simulink library "inp"  (Simulink 8.2)
settings: Solver=ode45  SolverMode=SingleTasking  StartTime=0.0  StopTime=10.0  RelTol=1e-3
undefined symbols (not defined in the file or in its folder): C_TICK_TIME_S, ENUM_FAULTSTATE_FAIL, ENUM_FAULTSTATE_INDETERMINATE, ENUM_FAULTSTATE_PASS, ENUM_KEYSWITCH_ON, FALSE, KINP_ABS1CANCommuOk_flg_ovrdflg, KINP_ABS1CANCommuOk_flg_ovrdval, KINP_ABS1CANPeriodTime_s, KINP_ABS1CANRolCntOk_flg_ovrdflg, KINP_ABS1CANRolCntOk_flg_ovrdval, KINP_ABS2CANChkSumOk_flg_ovrdflg, KINP_ABS2CANChkSumOk_flg_ovrdval, KINP_ABS2CANCommuOk_flg_ovrdflg, KINP_ABS2CANCommuOk_flg_ovrdval, KINP_ABS2CANPeriodTime_s, KINP_ABS2CANRolCntOk_flg_ovrdflg, KINP_ABS2CANRolCntOk_flg_ovrdval, KINP_ABS3CANCommuOk_flg_ovrdflg, KINP_ABS3CANCommuOk_flg_ovrdval, KINP_ABS4CANCommuOk_flg_ovrdflg, KINP_ABS4CANCommuOk_flg_ovrdval, KINP_ABSActive_flg_defval, KINP_ABSActive_flg_invalid, KINP_ABSActive_flg_ovrdflg, KINP_ABSActive_flg_ovrdval, KINP_ABSActive_flg_setvalid, KINP_ABSActive_flg_srvcflg, KINP_ABSActive_flg_srvcval, KINP_ABSCANAlive_flg_ovrdflg, KINP_ABSCANAlive_flg_ovrdval, KINP_ABSCANCommuOk_flg_ovrdflg, KINP_ABSCANCommuOk_flg_ovrdval, KINP_ABSCANValidOk_flg_ovrdflg, KINP_ABSCANValidOk_flg_ovrdval, KINP_ABSFault_flg_defval, KINP_ABSFault_flg_invalid, KINP_ABSFault_flg_ovrdflg, KINP_ABSFault_flg_ovrdval, KINP_ABSFault_flg_setvalid, KINP_ABSFault_flg_srvcflg, KINP_ABSFault_flg_srvcval, KINP_AC1CANCommuOk_flg_ovrdflg, KINP_AC1CANCommuOk_flg_ovrdval, KINP_ACCANAlive_flg_ovrdflg, KINP_ACCANAlive_flg_ovrdval, KINP_ACCANCommuOk_flg_ovrdflg, KINP_ACCANCommuOk_flg_ovrdval, KINP_ACCANValidOk_flg_ovrdflg, KINP_ACCANValidOk_flg_ovrdval, KINP_ACCCancelH_flg_defval, KINP_ACCCancelH_flg_invalid, KINP_ACCCancelH_flg_ovrdflg, KINP_ACCCancelH_flg_ovrdval, KINP_ACCCancelH_flg_setvalid, KINP_ACCCancelH_flg_srvcflg, KINP_ACCCancelH_flg_srvcval, KINP_AccelPedalPosn_pct_defval, KINP_AccelPedalPosn_pct_gain, KINP_AccelPedalPosn_pct_invalid ... (+1866)
libraries: inp x192, hcu_lib x34, simulink x33
(part 8 of 21)

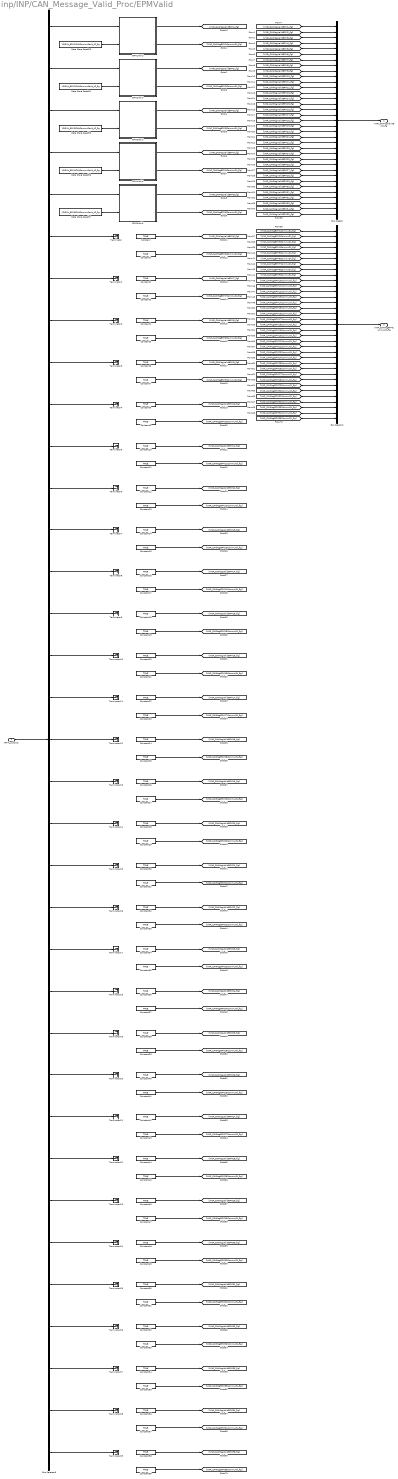
== system inp/INP/CAN_Message_Valid_Proc/EPMValid ==
  BHAL_outputs : Inport
  Bus Creator : BusCreator  Inputs=35
  Bus Creator1 : BusCreator  Inputs=35
  Bus Selector5 : BusSelector  OutputSignals=VHAL_CANMsgValidEPM1_flg,VHAL_CANMsgValidEPM2_flg,VHAL_CANMsgValidEPM3_flg,VHAL_CANMsgValidEPM4_flg,VHAL_CANMsgValidEPM5_flg,VHAL_CANMsgValidEPM6_flg,VHAL_CANMsgValidEPM7_flg,VHAL_CANMsgValidEPM8_flg,VHAL_CANMsgValidEPM9_flg,VHAL_CANMsgValidEPM10_flg,VHAL_CANMsgValidEPM11_flg,VHAL_CANMsgValidEPM12_flg,VHAL_CANMsgValidEPM13_flg,VHAL_CANMsgValidEPM14_flg,VHAL_CANMsgValidEPM15_flg,VHAL_CANMsgValidEPM16_flg,VHAL_CANMsgValidEPM17_flg,VHAL_CANMsgValidEPM18_flg,VHAL_CANMsgValidEPM19_flg,VHAL_CANMsgValidEPM20_flg,VHAL_CANMsgValidEPM21_flg,VHAL_CANMsgValidEPM22_flg,VHAL_CANMsgValidEPM23_flg,VHAL_CANMsgValidEPM24_flg,VHAL_CANMsgValidEPM25_flg,VHAL_CANMsgValidEPM26_flg,VHAL_CANMsgValidEPM27_flg,VHAL_CANMsgValidEPM28_flg,VHAL_CANMsgValidEPM29_flg,VHAL_CANMsgValidEPM30_flg,VHAL_CANMsgValidEPM31_flg,VHAL_CANMsgValidEPM32_flg,VHAL_CANMsgValidEPM33_flg,VHAL_CANMsgValidEPM34_flg,VHAL_CANMsgValidEPM35_flg
  Constant{,1..59} (60 alike) : Constant  Value=TRUE
  Data Store Read53 : DataStoreRead  DataStoreName=VDDML_EPM1CANCommuFault_AF_flg
  Data Store Read54 : DataStoreRead  DataStoreName=VDDML_EPM2CANCommuFault_AF_flg
  Data Store Read55 : DataStoreRead  DataStoreName=VDDML_EPM3CANCommuFault_AF_flg
  Data Store Read56 : DataStoreRead  DataStoreName=VDDML_EPM4CANCommuFault_AF_flg
  Data Store Read57 : DataStoreRead  DataStoreName=VDDML_EPM5CANCommuFault_AF_flg
  EPM1Valid : Subsystem
      settings: RTWSystemCode=Inline
  EPM2Valid : Subsystem
      settings: RTWSystemCode=Inline
  EPM3Valid : Subsystem
      settings: RTWSystemCode=Inline
  EPM4Valid : Subsystem
      settings: RTWSystemCode=Inline
  EPM5Valid : Subsystem
      settings: RTWSystemCode=Inline
  Terminator{,1..29} (30 alike) : Terminator
  VINP_CANMsgValidEPM_flg : Outport
  VINP_CANMsgEPMCommuOk_flg : Outport  Port=2
  wires:
  Data Store Read53/1 -> EPM1Valid/2(Active_fault_flg)
  Data Store Read54/1 -> EPM2Valid/2(Active_fault_flg)
  Data Store Read55/1 -> EPM3Valid/2(Active_fault_flg)
  Data Store Read56/1 -> EPM4Valid/2(Active_fault_flg)
  Data Store Read57/1 -> EPM5Valid/2(Active_fault_flg)
  EPM1Valid/1(signal_out) -> [VINP_CANMsgValidEPM1_flg]  "VINP_CANMsgValidEPM1_flg"
  EPM1Valid/2(signal_valid_out) -> [VINP_CANMsgEPM1CommuOk_flg]  "VINP_CANMsgEPM1CommuOk_flg"
  EPM2Valid/1(signal_out) -> [VINP_CANMsgValidEPM2_flg]  "VINP_CANMsgValidEPM2_flg"
  EPM2Valid/2(signal_valid_out) -> [VINP_CANMsgEPM2CommuOk_flg]  "VINP_CANMsgEPM2CommuOk_flg"
  EPM3Valid/1(signal_out) -> [VINP_CANMsgValidEPM3_flg]  "VINP_CANMsgValidEPM3_flg"
  EPM3Valid/2(signal_valid_out) -> [VINP_CANMsgEPM3CommuOk_flg]  "VINP_CANMsgEPM3CommuOk_flg"
  EPM4Valid/1(signal_out) -> [VINP_CANMsgValidEPM4_flg]  "VINP_CANMsgValidEPM4_flg"
  EPM4Valid/2(signal_valid_out) -> [VINP_CANMsgEPM4CommuOk_flg]  "VINP_CANMsgEPM4CommuOk_flg"
  EPM5Valid/1(signal_out) -> [VINP_CANMsgValidEPM5_flg]  "VINP_CANMsgValidEPM5_flg"
  EPM5Valid/2(signal_valid_out) -> [VINP_CANMsgEPM5CommuOk_flg]  "VINP_CANMsgEPM5CommuOk_flg"
  BHAL_outputs/1 -> Bus Selector5/1
  Bus Selector5/1 -> EPM1Valid/1(signal_in)  "<VHAL_CANMsgValidEPM1_flg>"
  Bus Selector5/2 -> EPM2Valid/1(signal_in)  "<VHAL_CANMsgValidEPM2_flg>"
  Bus Selector5/3 -> EPM3Valid/1(signal_in)  "<VHAL_CANMsgValidEPM3_flg>"
  Bus Selector5/4 -> EPM4Valid/1(signal_in)  "<VHAL_CANMsgValidEPM4_flg>"
  Bus Selector5/5 -> EPM5Valid/1(signal_in)  "<VHAL_CANMsgValidEPM5_flg>"
  Bus Selector5/6 -> Terminator/1  "<VHAL_CANMsgValidEPM6_flg>"
  Constant/1 -> [VINP_CANMsgValidEPM6_flg]  "VINP_CANMsgValidEPM6_flg"
  Constant1/1 -> [VINP_CANMsgEPM6CommuOk_flg]  "VINP_CANMsgEPM6CommuOk_flg"
  Constant2/1 -> [VINP_CANMsgValidEPM7_flg]  "VINP_CANMsgValidEPM7_flg"
  Constant3/1 -> [VINP_CANMsgEPM7CommuOk_flg]  "VINP_CANMsgEPM7CommuOk_flg"
  Bus Selector5/7 -> Terminator1/1  "<VHAL_CANMsgValidEPM7_flg>"
  Constant4/1 -> [VINP_CANMsgValidEPM8_flg]  "VINP_CANMsgValidEPM8_flg"
  Constant5/1 -> [VINP_CANMsgEPM8CommuOk_flg]  "VINP_CANMsgEPM8CommuOk_flg"
  Bus Selector5/8 -> Terminator2/1  "<VHAL_CANMsgValidEPM8_flg>"
  Constant6/1 -> [VINP_CANMsgValidEPM9_flg]  "VINP_CANMsgValidEPM9_flg"
  Constant7/1 -> [VINP_CANMsgEPM9CommuOk_flg]  "VINP_CANMsgEPM9CommuOk_flg"
  Bus Selector5/9 -> Terminator3/1  "<VHAL_CANMsgValidEPM9_flg>"
  Constant8/1 -> [VINP_CANMsgValidEPM10_flg]  "VINP_CANMsgValidEPM10_flg"
  Constant9/1 -> [VINP_CANMsgEPM10CommuOk_flg]  "VINP_CANMsgEPM10CommuOk_flg"
  Bus Selector5/10 -> Terminator4/1  "<VHAL_CANMsgValidEPM10_flg>"
  Constant10/1 -> [VINP_CANMsgValidEPM11_flg]  "VINP_CANMsgValidEPM11_flg"
  Constant11/1 -> [VINP_CANMsgEPM11CommuOk_flg]  "VINP_CANMsgEPM11CommuOk_flg"
  Bus Selector5/11 -> Terminator5/1  "<VHAL_CANMsgValidEPM11_flg>"
  Constant12/1 -> [VINP_CANMsgValidEPM12_flg]  "VINP_CANMsgValidEPM12_flg"
  Constant13/1 -> [VINP_CANMsgEPM12CommuOk_flg]  "VINP_CANMsgEPM12CommuOk_flg"
  Bus Selector5/12 -> Terminator6/1  "<VHAL_CANMsgValidEPM12_flg>"
  Constant14/1 -> [VINP_CANMsgValidEPM13_flg]  "VINP_CANMsgValidEPM13_flg"
  Constant15/1 -> [VINP_CANMsgEPM13CommuOk_flg]  "VINP_CANMsgEPM13CommuOk_flg"
  Bus Selector5/13 -> Terminator7/1  "<VHAL_CANMsgValidEPM13_flg>"
  Constant16/1 -> [VINP_CANMsgValidEPM14_flg]  "VINP_CANMsgValidEPM14_flg"
  Constant17/1 -> [VINP_CANMsgEPM14CommuOk_flg]  "VINP_CANMsgEPM14CommuOk_flg"
  Bus Selector5/14 -> Terminator8/1  "<VHAL_CANMsgValidEPM14_flg>"
  Constant18/1 -> [VINP_CANMsgValidEPM15_flg]  "VINP_CANMsgValidEPM15_flg"
  Constant19/1 -> [VINP_CANMsgEPM15CommuOk_flg]  "VINP_CANMsgEPM15CommuOk_flg"
  Bus Selector5/15 -> Terminator9/1  "<VHAL_CANMsgValidEPM15_flg>"
  Constant20/1 -> [VINP_CANMsgValidEPM16_flg]  "VINP_CANMsgValidEPM16_flg"
  Constant21/1 -> [VINP_CANMsgEPM16CommuOk_flg]  "VINP_CANMsgEPM16CommuOk_flg"
  Bus Selector5/16 -> Terminator10/1  "<VHAL_CANMsgValidEPM16_flg>"
  Constant22/1 -> [VINP_CANMsgValidEPM17_flg]  "VINP_CANMsgValidEPM17_flg"
  Constant23/1 -> [VINP_CANMsgEPM17CommuOk_flg]  "VINP_CANMsgEPM17CommuOk_flg"
  Bus Selector5/17 -> Terminator11/1  "<VHAL_CANMsgValidEPM17_flg>"
  Constant24/1 -> [VINP_CANMsgValidEPM18_flg]  "VINP_CANMsgValidEPM18_flg"
  Constant25/1 -> [VINP_CANMsgEPM18CommuOk_flg]  "VINP_CANMsgEPM18CommuOk_flg"
  Bus Selector5/18 -> Terminator12/1  "<VHAL_CANMsgValidEPM18_flg>"
  Constant26/1 -> [VINP_CANMsgValidEPM19_flg]  "VINP_CANMsgValidEPM19_flg"
  Constant27/1 -> [VINP_CANMsgEPM19CommuOk_flg]  "VINP_CANMsgEPM19CommuOk_flg"
  Bus Selector5/19 -> Terminator13/1  "<VHAL_CANMsgValidEPM19_flg>"
  Constant28/1 -> [VINP_CANMsgValidEPM20_flg]  "VINP_CANMsgValidEPM20_flg"
  Constant29/1 -> [VINP_CANMsgEPM20CommuOk_flg]  "VINP_CANMsgEPM20CommuOk_flg"
  Bus Selector5/20 -> Terminator14/1  "<VHAL_CANMsgValidEPM20_flg>"
  Constant30/1 -> [VINP_CANMsgValidEPM21_flg]  "VINP_CANMsgValidEPM21_flg"
  Constant31/1 -> [VINP_CANMsgEPM21CommuOk_flg]  "VINP_CANMsgEPM21CommuOk_flg"
  Bus Selector5/21 -> Terminator15/1  "<VHAL_CANMsgValidEPM21_flg>"
  Constant32/1 -> [VINP_CANMsgValidEPM22_flg]  "VINP_CANMsgValidEPM22_flg"
  Constant33/1 -> [VINP_CANMsgEPM22CommuOk_flg]  "VINP_CANMsgEPM22CommuOk_flg"
  Bus Selector5/22 -> Terminator16/1  "<VHAL_CANMsgValidEPM22_flg>"
  Constant34/1 -> [VINP_CANMsgValidEPM23_flg]  "VINP_CANMsgValidEPM23_flg"
  Constant35/1 -> [VINP_CANMsgEPM23CommuOk_flg]  "VINP_CANMsgEPM23CommuOk_flg"
  Bus Selector5/23 -> Terminator17/1  "<VHAL_CANMsgValidEPM23_flg>"
  Constant36/1 -> [VINP_CANMsgValidEPM24_flg]  "VINP_CANMsgValidEPM24_flg"
  Constant37/1 -> [VINP_CANMsgEPM24CommuOk_flg]  "VINP_CANMsgEPM24CommuOk_flg"
  Bus Selector5/24 -> Terminator18/1  "<VHAL_CANMsgValidEPM24_flg>"
  Constant38/1 -> [VINP_CANMsgValidEPM25_flg]  "VINP_CANMsgValidEPM25_flg"
  Constant39/1 -> [VINP_CANMsgEPM25CommuOk_flg]  "VINP_CANMsgEPM25CommuOk_flg"
  Bus Selector5/25 -> Terminator19/1  "<VHAL_CANMsgValidEPM25_flg>"
  Constant40/1 -> [VINP_CANMsgValidEPM26_flg]  "VINP_CANMsgValidEPM26_flg"
  Constant41/1 -> [VINP_CANMsgEPM26CommuOk_flg]  "VINP_CANMsgEPM26CommuOk_flg"
  Bus Selector5/26 -> Terminator20/1  "<VHAL_CANMsgValidEPM26_flg>"
  Constant42/1 -> [VINP_CANMsgValidEPM27_flg]  "VINP_CANMsgValidEPM27_flg"
  Constant43/1 -> [VINP_CANMsgEPM27CommuOk_flg]  "VINP_CANMsgEPM27CommuOk_flg"
  Bus Selector5/27 -> Terminator21/1  "<VHAL_CANMsgValidEPM27_flg>"
  Constant44/1 -> [VINP_CANMsgValidEPM28_flg]  "VINP_CANMsgValidEPM28_flg"
  Constant45/1 -> [VINP_CANMsgEPM28CommuOk_flg]  "VINP_CANMsgEPM28CommuOk_flg"
  Bus Selector5/28 -> Terminator22/1  "<VHAL_CANMsgValidEPM28_flg>"
  Constant46/1 -> [VINP_CANMsgValidEPM29_flg]  "VINP_CANMsgValidEPM29_flg"
  Constant47/1 -> [VINP_CANMsgEPM29CommuOk_flg]  "VINP_CANMsgEPM29CommuOk_flg"
  Bus Selector5/29 -> Terminator23/1  "<VHAL_CANMsgValidEPM29_flg>"
  Constant48/1 -> [VINP_CANMsgValidEPM30_flg]  "VINP_CANMsgValidEPM30_flg"
  Constant49/1 -> [VINP_CANMsgEPM30CommuOk_flg]  "VINP_CANMsgEPM30CommuOk_flg"
  Bus Selector5/30 -> Terminator24/1  "<VHAL_CANMsgValidEPM30_flg>"
  Constant50/1 -> [VINP_CANMsgValidEPM31_flg]  "VINP_CANMsgValidEPM31_flg"
  Constant51/1 -> [VINP_CANMsgEPM31CommuOk_flg]  "VINP_CANMsgEPM31CommuOk_flg"
  Bus Selector5/31 -> Terminator25/1  "<VHAL_CANMsgValidEPM31_flg>"
  Constant52/1 -> [VINP_CANMsgValidEPM32_flg]  "VINP_CANMsgValidEPM32_flg"
  Constant53/1 -> [VINP_CANMsgEPM32CommuOk_flg]  "VINP_CANMsgEPM32CommuOk_flg"
  Bus Selector5/32 -> Terminator26/1  "<VHAL_CANMsgValidEPM32_flg>"
  Constant54/1 -> [VINP_CANMsgValidEPM33_flg]  "VINP_CANMsgValidEPM33_flg"
  Constant55/1 -> [VINP_CANMsgEPM33CommuOk_flg]  "VINP_CANMsgEPM33CommuOk_flg"
  Bus Selector5/33 -> Terminator27/1  "<VHAL_CANMsgValidEPM33_flg>"
  Constant56/1 -> [VINP_CANMsgValidEPM34_flg]  "VINP_CANMsgValidEPM34_flg"
  Constant57/1 -> [VINP_CANMsgEPM34CommuOk_flg]  "VINP_CANMsgEPM34CommuOk_flg"
  Bus Selector5/34 -> Terminator28/1  "<VHAL_CANMsgValidEPM34_flg>"
  Constant58/1 -> [VINP_CANMsgValidEPM35_flg]  "VINP_CANMsgValidEPM35_flg"
  Constant59/1 -> [VINP_CANMsgEPM35CommuOk_flg]  "VINP_CANMsgEPM35CommuOk_flg"
  Bus Selector5/35 -> Terminator29/1  "<VHAL_CANMsgValidEPM35_flg>"
  [VINP_CANMsgValidEPM8_flg] -> Bus Creator/8
  [VINP_CANMsgValidEPM9_flg] -> Bus Creator/9
  [VINP_CANMsgValidEPM4_flg] -> Bus Creator/4
  [VINP_CANMsgValidEPM3_flg] -> Bus Creator/3
  [VINP_CANMsgValidEPM2_flg] -> Bus Creator/2
  [VINP_CANMsgValidEPM7_flg] -> Bus Creator/7
  [VINP_CANMsgValidEPM1_flg] -> Bus Creator/1
  [VINP_CANMsgValidEPM5_flg] -> Bus Creator/5
  [VINP_CANMsgValidEPM6_flg] -> Bus Creator/6
  [VINP_CANMsgValidEPM10_flg] -> Bus Creator/10
  [VINP_CANMsgValidEPM12_flg] -> Bus Creator/12
  [VINP_CANMsgValidEPM16_flg] -> Bus Creator/16
  [VINP_CANMsgValidEPM11_flg] -> Bus Creator/11
  [VINP_CANMsgValidEPM14_flg] -> Bus Creator/14
  [VINP_CANMsgValidEPM15_flg] -> Bus Creator/15
  [VINP_CANMsgValidEPM13_flg] -> Bus Creator/13
  [VINP_CANMsgValidEPM28_flg] -> Bus Creator/28
  [VINP_CANMsgValidEPM19_flg] -> Bus Creator/19
  [VINP_CANMsgValidEPM35_flg] -> Bus Creator/35
  [VINP_CANMsgValidEPM18_flg] -> Bus Creator/18
  [VINP_CANMsgValidEPM26_flg] -> Bus Creator/26
  [VINP_CANMsgValidEPM17_flg] -> Bus Creator/17
  [VINP_CANMsgValidEPM22_flg] -> Bus Creator/22
  [VINP_CANMsgValidEPM20_flg] -> Bus Creator/20
  [VINP_CANMsgValidEPM25_flg] -> Bus Creator/25
  [VINP_CANMsgValidEPM23_flg] -> Bus Creator/23
  [VINP_CANMsgValidEPM29_flg] -> Bus Creator/29
  [VINP_CANMsgValidEPM21_flg] -> Bus Creator/21
  [VINP_CANMsgValidEPM24_flg] -> Bus Creator/24
  [VINP_CANMsgValidEPM31_flg] -> Bus Creator/31
  [VINP_CANMsgValidEPM27_flg] -> Bus Creator/27
  [VINP_CANMsgValidEPM30_flg] -> Bus Creator/30
  [VINP_CANMsgValidEPM32_flg] -> Bus Creator/32
  [VINP_CANMsgValidEPM33_flg] -> Bus Creator/33
  [VINP_CANMsgValidEPM34_flg] -> Bus Creator/34
  Bus Creator/1 -> VINP_CANMsgValidEPM_flg/1
  Bus Creator1/1 -> VINP_CANMsgEPMCommuOk_flg/1
  [VINP_CANMsgEPM25CommuOk_flg] -> Bus Creator1/25
  [VINP_CANMsgEPM27CommuOk_flg] -> Bus Creator1/27
  [VINP_CANMsgEPM22CommuOk_flg] -> Bus Creator1/22
  [VINP_CANMsgEPM26CommuOk_flg] -> Bus Creator1/26
  [VINP_CANMsgEPM8CommuOk_flg] -> Bus Creator1/8
  [VINP_CANMsgEPM3CommuOk_flg] -> Bus Creator1/3
  [VINP_CANMsgEPM1CommuOk_flg] -> Bus Creator1/1
  [VINP_CANMsgEPM4CommuOk_flg] -> Bus Creator1/4
  [VINP_CANMsgEPM18CommuOk_flg] -> Bus Creator1/18
  [VINP_CANMsgEPM5CommuOk_flg] -> Bus Creator1/5
  [VINP_CANMsgEPM2CommuOk_flg] -> Bus Creator1/2
  [VINP_CANMsgEPM12CommuOk_flg] -> Bus Creator1/12
  [VINP_CANMsgEPM7CommuOk_flg] -> Bus Creator1/7
  [VINP_CANMsgEPM21CommuOk_flg] -> Bus Creator1/21
  [VINP_CANMsgEPM15CommuOk_flg] -> Bus Creator1/15
  [VINP_CANMsgEPM16CommuOk_flg] -> Bus Creator1/16
  [VINP_CANMsgEPM6CommuOk_flg] -> Bus Creator1/6
  [VINP_CANMsgEPM9CommuOk_flg] -> Bus Creator1/9
  [VINP_CANMsgEPM23CommuOk_flg] -> Bus Creator1/23
  [VINP_CANMsgEPM10CommuOk_flg] -> Bus Creator1/10
  [VINP_CANMsgEPM11CommuOk_flg] -> Bus Creator1/11
  [VINP_CANMsgEPM13CommuOk_flg] -> Bus Creator1/13
  [VINP_CANMsgEPM24CommuOk_flg] -> Bus Creator1/24
  [VINP_CANMsgEPM14CommuOk_flg] -> Bus Creator1/14
  [VINP_CANMsgEPM20CommuOk_flg] -> Bus Creator1/20
  [VINP_CANMsgEPM19CommuOk_flg] -> Bus Creator1/19
  [VINP_CANMsgEPM17CommuOk_flg] -> Bus Creator1/17
  [VINP_CANMsgEPM28CommuOk_flg] -> Bus Creator1/28
  [VINP_CANMsgEPM34CommuOk_flg] -> Bus Creator1/34
  [VINP_CANMsgEPM29CommuOk_flg] -> Bus Creator1/29
  [VINP_CANMsgEPM35CommuOk_flg] -> Bus Creator1/35
  [VINP_CANMsgEPM33CommuOk_flg] -> Bus Creator1/33
  [VINP_CANMsgEPM30CommuOk_flg] -> Bus Creator1/30
  [VINP_CANMsgEPM31CommuOk_flg] -> Bus Creator1/31
  [VINP_CANMsgEPM32CommuOk_flg] -> Bus Creator1/32

== system inp/INP/CAN_Message_Valid_Proc/HCU3Updated ==
  signal_in : Inport
  diagnosis_switch : Inport  Port=2
  Active_fault_flg : Inport  Port=3
  can_valid_process : inp/can_valid_process
  defval : Constant  Value=KINP_CANMsgUpdatedHCU3_flg_defval
  invalid : Constant  Value=KINP_CANMsgUpdatedHCU3_flg_invalid
  ovrdflg : Constant  Value=KINP_CANMsgUpdatedHCU3_flg_ovrdflg
  ovrdval : Constant  Value=KINP_CANMsgUpdatedHCU3_flg_ovrdval
  setvalid : Constant  Value=KINP_CANMsgUpdatedHCU3_flg_setvalid
  srvcflg : Constant  Value=KINP_CANMsgUpdatedHCU3_flg_srvcflg
  srvcval : Constant  Value=KINP_CANMsgUpdatedHCU3_flg_srvcval
  tempval : Constant  Value=KINP_CANMsgUpdatedHCU3_flg_tempval
  signal_out : Outport
  signal_valid_out : Outport  Port=2
  wires:
  srvcval/1 -> can_valid_process/11
  srvcflg/1 -> can_valid_process/10
  ovrdval/1 -> can_valid_process/9
  ovrdflg/1 -> can_valid_process/8
  setvalid/1 -> can_valid_process/7
  invalid/1 -> can_valid_process/6
  tempval/1 -> can_valid_process/5
  defval/1 -> can_valid_process/4
  diagnosis_switch/1 -> can_valid_process/3
  Active_fault_flg/1 -> can_valid_process/2
  signal_in/1 -> can_valid_process/1
  can_valid_process/2 -> signal_valid_out/1
  can_valid_process/1 -> signal_out/1

== system inp/INP/CAN_Message_Valid_Proc/HCU3Valid ==
  signal_in : Inport
  Active_fault_flg : Inport  Port=2
  TRUE : Constant  Value=TRUE
  can_valid_process : inp/can_valid_process
  defval : Constant  Value=KINP_CANMsgValidHCU3_flg_defval
  invalid : Constant  Value=KINP_CANMsgValidHCU3_flg_invalid
  ovrdflg : Constant  Value=KINP_CANMsgValidHCU3_flg_ovrdflg
  ovrdval : Constant  Value=KINP_CANMsgValidHCU3_flg_ovrdval
  setvalid : Constant  Value=KINP_CANMsgValidHCU3_flg_setvalid
  srvcflg : Constant  Value=KINP_CANMsgValidHCU3_flg_srvcflg
  srvcval : Constant  Value=KINP_CANMsgValidHCU3_flg_srvcval
  tempval : Constant  Value=KINP_CANMsgValidHCU3_flg_tempval
  signal_out : Outport
  signal_valid_out : Outport  Port=2
  wires:
  srvcval/1 -> can_valid_process/11
  srvcflg/1 -> can_valid_process/10
  ovrdval/1 -> can_valid_process/9
  ovrdflg/1 -> can_valid_process/8
  setvalid/1 -> can_valid_process/7
  invalid/1 -> can_valid_process/6
  tempval/1 -> can_valid_process/5
  defval/1 -> can_valid_process/4
  TRUE/1 -> can_valid_process/3
  Active_fault_flg/1 -> can_valid_process/2
  signal_in/1 -> can_valid_process/1
  can_valid_process/2 -> signal_valid_out/1
  can_valid_process/1 -> signal_out/1

== system inp/INP/CAN_Message_Valid_Proc/HCU4Valid ==
  signal_in : Inport
  Active_fault_flg : Inport  Port=2
  TRUE : Constant  Value=TRUE
  can_valid_process : inp/can_valid_process
  defval : Constant  Value=KINP_CANMsgValidHCU4_flg_defval
  invalid : Constant  Value=KINP_CANMsgValidHCU4_flg_invalid
  ovrdflg : Constant  Value=KINP_CANMsgValidHCU4_flg_ovrdflg
  ovrdval : Constant  Value=KINP_CANMsgValidHCU4_flg_ovrdval
  setvalid : Constant  Value=KINP_CANMsgValidHCU4_flg_setvalid
  srvcflg : Constant  Value=KINP_CANMsgValidHCU4_flg_srvcflg
  srvcval : Constant  Value=KINP_CANMsgValidHCU4_flg_srvcval
  tempval : Constant  Value=KINP_CANMsgValidHCU4_flg_tempval
  signal_out : Outport
  signal_valid_out : Outport  Port=2
  wires:
  can_valid_process/1 -> signal_out/1
  can_valid_process/2 -> signal_valid_out/1
  signal_in/1 -> can_valid_process/1
  Active_fault_flg/1 -> can_valid_process/2
  TRUE/1 -> can_valid_process/3
  defval/1 -> can_valid_process/4
  tempval/1 -> can_valid_process/5
  invalid/1 -> can_valid_process/6
  setvalid/1 -> can_valid_process/7
  ovrdflg/1 -> can_valid_process/8
  ovrdval/1 -> can_valid_process/9
  srvcflg/1 -> can_valid_process/10
  srvcval/1 -> can_valid_process/11

== system inp/INP/CAN_Message_Valid_Proc/HMI1Valid ==
  signal_in : Inport
  Active_fault_flg : Inport  Port=2
  TRUE : Constant  Value=TRUE
  can_valid_process : inp/can_valid_process
  defval : Constant  Value=KINP_CANMsgValidHMI1_flg_defval
  invalid : Constant  Value=KINP_CANMsgValidHMI1_flg_invalid
  ovrdflg : Constant  Value=KINP_CANMsgValidHMI1_flg_ovrdflg
  ovrdval : Constant  Value=KINP_CANMsgValidHMI1_flg_ovrdval
  setvalid : Constant  Value=KINP_CANMsgValidHMI1_flg_setvalid
  srvcflg : Constant  Value=KINP_CANMsgValidHMI1_flg_srvcflg
  srvcval : Constant  Value=KINP_CANMsgValidHMI1_flg_srvcval
  tempval : Constant  Value=KINP_CANMsgValidHMI1_flg_tempval
  signal_out : Outport
  signal_valid_out : Outport  Port=2
  wires:
  srvcval/1 -> can_valid_process/11
  srvcflg/1 -> can_valid_process/10
  ovrdval/1 -> can_valid_process/9
  ovrdflg/1 -> can_valid_process/8
  setvalid/1 -> can_valid_process/7
  invalid/1 -> can_valid_process/6
  tempval/1 -> can_valid_process/5
  defval/1 -> can_valid_process/4
  TRUE/1 -> can_valid_process/3
  Active_fault_flg/1 -> can_valid_process/2
  signal_in/1 -> can_valid_process/1
  can_valid_process/2 -> signal_valid_out/1
  can_valid_process/1 -> signal_out/1

== system inp/INP/CAN_Message_Valid_Proc/HMI2Valid ==
  signal_in : Inport
  Active_fault_flg : Inport  Port=2
  TRUE : Constant  Value=TRUE
  can_valid_process : inp/can_valid_process
  defval : Constant  Value=KINP_CANMsgValidHMI2_flg_defval
  invalid : Constant  Value=KINP_CANMsgValidHMI2_flg_invalid
  ovrdflg : Constant  Value=KINP_CANMsgValidHMI2_flg_ovrdflg
  ovrdval : Constant  Value=KINP_CANMsgValidHMI2_flg_ovrdval
  setvalid : Constant  Value=KINP_CANMsgValidHMI2_flg_setvalid
  srvcflg : Constant  Value=KINP_CANMsgValidHMI2_flg_srvcflg
  srvcval : Constant  Value=KINP_CANMsgValidHMI2_flg_srvcval
  tempval : Constant  Value=KINP_CANMsgValidHMI2_flg_tempval
  signal_out : Outport
  signal_valid_out : Outport  Port=2
  wires:
  can_valid_process/1 -> signal_out/1
  can_valid_process/2 -> signal_valid_out/1
  signal_in/1 -> can_valid_process/1
  Active_fault_flg/1 -> can_valid_process/2
  TRUE/1 -> can_valid_process/3
  defval/1 -> can_valid_process/4
  tempval/1 -> can_valid_process/5
  invalid/1 -> can_valid_process/6
  setvalid/1 -> can_valid_process/7
  ovrdflg/1 -> can_valid_process/8
  ovrdval/1 -> can_valid_process/9
  srvcflg/1 -> can_valid_process/10
  srvcval/1 -> can_valid_process/11

== system inp/INP/CAN_Message_Valid_Proc/METER1Valid ==
  signal_in : Inport
  Active_fault_flg : Inport  Port=2
  TRUE : Constant  Value=TRUE
  can_valid_process : inp/can_valid_process
  defval : Constant  Value=KINP_CANMsgValidMETER1_flg_defval
  invalid : Constant  Value=KINP_CANMsgValidMETER1_flg_invalid
  ovrdflg : Constant  Value=KINP_CANMsgValidMETER1_flg_ovrdflg
  ovrdval : Constant  Value=KINP_CANMsgValidMETER1_flg_ovrdval
  setvalid : Constant  Value=KINP_CANMsgValidMETER1_flg_setvalid
  srvcflg : Constant  Value=KINP_CANMsgValidMETER1_flg_srvcflg
  srvcval : Constant  Value=KINP_CANMsgValidMETER1_flg_srvcval
  tempval : Constant  Value=KINP_CANMsgValidMETER1_flg_tempval
  signal_out : Outport
  signal_valid_out : Outport  Port=2
  wires:
  srvcval/1 -> can_valid_process/11
  srvcflg/1 -> can_valid_process/10
  ovrdval/1 -> can_valid_process/9
  ovrdflg/1 -> can_valid_process/8
  setvalid/1 -> can_valid_process/7
  invalid/1 -> can_valid_process/6
  tempval/1 -> can_valid_process/5
  defval/1 -> can_valid_process/4
  TRUE/1 -> can_valid_process/3
  Active_fault_flg/1 -> can_valid_process/2
  signal_in/1 -> can_valid_process/1
  can_valid_process/2 -> signal_valid_out/1
  can_valid_process/1 -> signal_out/1

== system inp/INP/CAN_Message_Valid_Proc/METER3Valid ==
  signal_in : Inport
  Active_fault_flg : Inport  Port=2
  TRUE : Constant  Value=TRUE
  can_valid_process : inp/can_valid_process
  defval : Constant  Value=KINP_CANMsgValidMETER3_flg_defval
  invalid : Constant  Value=KINP_CANMsgValidMETER3_flg_invalid
  ovrdflg : Constant  Value=KINP_CANMsgValidMETER3_flg_ovrdflg
  ovrdval : Constant  Value=KINP_CANMsgValidMETER3_flg_ovrdval
  setvalid : Constant  Value=KINP_CANMsgValidMETER3_flg_setvalid
  srvcflg : Constant  Value=KINP_CANMsgValidMETER3_flg_srvcflg
  srvcval : Constant  Value=KINP_CANMsgValidMETER3_flg_srvcval
  tempval : Constant  Value=KINP_CANMsgValidMETER3_flg_tempval
  signal_out : Outport
  signal_valid_out : Outport  Port=2
  wires:
  can_valid_process/1 -> signal_out/1
  can_valid_process/2 -> signal_valid_out/1
  signal_in/1 -> can_valid_process/1
  Active_fault_flg/1 -> can_valid_process/2
  TRUE/1 -> can_valid_process/3
  defval/1 -> can_valid_process/4
  tempval/1 -> can_valid_process/5
  invalid/1 -> can_valid_process/6
  setvalid/1 -> can_valid_process/7
  ovrdflg/1 -> can_valid_process/8
  ovrdval/1 -> can_valid_process/9
  srvcflg/1 -> can_valid_process/10
  srvcval/1 -> can_valid_process/11

== system inp/INP/CAN_Message_Valid_Proc/SAS1Checked ==
  signal_in : Inport
  diagnosis_switch : Inport  Port=2
  Active_fault_flg : Inport  Port=3
  can_valid_process : inp/can_valid_process
  defval : Constant  Value=KINP_CANMsgCheckedSAS1_flg_defval
  invalid : Constant  Value=KINP_CANMsgCheckedSAS1_flg_invalid
  ovrdflg : Constant  Value=KINP_CANMsgCheckedSAS1_flg_ovrdflg
  ovrdval : Constant  Value=KINP_CANMsgCheckedSAS1_flg_ovrdval
  setvalid : Constant  Value=KINP_CANMsgCheckedSAS1_flg_setvalid
  srvcflg : Constant  Value=KINP_CANMsgCheckedSAS1_flg_srvcflg
  srvcval : Constant  Value=KINP_CANMsgCheckedSAS1_flg_srvcval
  tempval : Constant  Value=KINP_CANMsgCheckedSAS1_flg_tempval
  signal_out : Outport
  signal_valid_out : Outport  Port=2
  wires:
  srvcval/1 -> can_valid_process/11
  srvcflg/1 -> can_valid_process/10
  ovrdval/1 -> can_valid_process/9
  ovrdflg/1 -> can_valid_process/8
  setvalid/1 -> can_valid_process/7
  invalid/1 -> can_valid_process/6
  tempval/1 -> can_valid_process/5
  defval/1 -> can_valid_process/4
  diagnosis_switch/1 -> can_valid_process/3
  Active_fault_flg/1 -> can_valid_process/2
  signal_in/1 -> can_valid_process/1
  can_valid_process/2 -> signal_valid_out/1
  can_valid_process/1 -> signal_out/1

== system inp/INP/CAN_Message_Valid_Proc/SAS1Updated ==
  signal_in : Inport
  diagnosis_switch : Inport  Port=2
  Active_fault_flg : Inport  Port=3
  can_valid_process : inp/can_valid_process
  defval : Constant  Value=KINP_CANMsgUpdatedSAS1_flg_defval
  invalid : Constant  Value=KINP_CANMsgUpdatedSAS1_flg_invalid
  ovrdflg : Constant  Value=KINP_CANMsgUpdatedSAS1_flg_ovrdflg
  ovrdval : Constant  Value=KINP_CANMsgUpdatedSAS1_flg_ovrdval
  setvalid : Constant  Value=KINP_CANMsgUpdatedSAS1_flg_setvalid
  srvcflg : Constant  Value=KINP_CANMsgUpdatedSAS1_flg_srvcflg
  srvcval : Constant  Value=KINP_CANMsgUpdatedSAS1_flg_srvcval
  tempval : Constant  Value=KINP_CANMsgUpdatedSAS1_flg_tempval
  signal_out : Outport
  signal_valid_out : Outport  Port=2
  wires:
  can_valid_process/1 -> signal_out/1
  can_valid_process/2 -> signal_valid_out/1
  signal_in/1 -> can_valid_process/1
  Active_fault_flg/1 -> can_valid_process/2
  diagnosis_switch/1 -> can_valid_process/3
  defval/1 -> can_valid_process/4
  tempval/1 -> can_valid_process/5
  invalid/1 -> can_valid_process/6
  setvalid/1 -> can_valid_process/7
  ovrdflg/1 -> can_valid_process/8
  ovrdval/1 -> can_valid_process/9
  srvcflg/1 -> can_valid_process/10
  srvcval/1 -> can_valid_process/11

== system inp/INP/CAN_Message_Valid_Proc/SAS1Valid ==
  signal_in : Inport
  Active_fault_flg : Inport  Port=2
  TRUE : Constant  Value=TRUE
  can_valid_process : inp/can_valid_process
  defval : Constant  Value=KINP_CANMsgValidSAS1_flg_defval
  invalid : Constant  Value=KINP_CANMsgValidSAS1_flg_invalid
  ovrdflg : Constant  Value=KINP_CANMsgValidSAS1_flg_ovrdflg
  ovrdval : Constant  Value=KINP_CANMsgValidSAS1_flg_ovrdval
  setvalid : Constant  Value=KINP_CANMsgValidSAS1_flg_setvalid
  srvcflg : Constant  Value=KINP_CANMsgValidSAS1_flg_srvcflg
  srvcval : Constant  Value=KINP_CANMsgValidSAS1_flg_srvcval
  tempval : Constant  Value=KINP_CANMsgValidSAS1_flg_tempval
  signal_out : Outport
  signal_valid_out : Outport  Port=2
  wires:
  can_valid_process/1 -> signal_out/1
  can_valid_process/2 -> signal_valid_out/1
  signal_in/1 -> can_valid_process/1
  Active_fault_flg/1 -> can_valid_process/2
  TRUE/1 -> can_valid_process/3
  defval/1 -> can_valid_process/4
  tempval/1 -> can_valid_process/5
  invalid/1 -> can_valid_process/6
  setvalid/1 -> can_valid_process/7
  ovrdflg/1 -> can_valid_process/8
  ovrdval/1 -> can_valid_process/9
  srvcflg/1 -> can_valid_process/10
  srvcval/1 -> can_valid_process/11

== system inp/INP/CAN_Message_Valid_Proc/TCM1Valid ==
  signal_in : Inport
  Active_fault_flg : Inport  Port=2
  TRUE : Constant  Value=TRUE
  can_valid_process : inp/can_valid_process
  defval : Constant  Value=KINP_CANMsgValidTCM1_flg_defval
  invalid : Constant  Value=KINP_CANMsgValidTCM1_flg_invalid
  ovrdflg : Constant  Value=KINP_CANMsgValidTCM1_flg_ovrdflg
  ovrdval : Constant  Value=KINP_CANMsgValidTCM1_flg_ovrdval
  setvalid : Constant  Value=KINP_CANMsgValidTCM1_flg_setvalid
  srvcflg : Constant  Value=KINP_CANMsgValidTCM1_flg_srvcflg
  srvcval : Constant  Value=KINP_CANMsgValidTCM1_flg_srvcval
  tempval : Constant  Value=KINP_CANMsgValidTCM1_flg_tempval
  signal_out : Outport
  signal_valid_out : Outport  Port=2
  wires:
  Active_fault_flg/1 -> can_valid_process/2
  signal_in/1 -> can_valid_process/1
  can_valid_process/2 -> signal_valid_out/1
  can_valid_process/1 -> signal_out/1
  TRUE/1 -> can_valid_process/3
  defval/1 -> can_valid_process/4
  tempval/1 -> can_valid_process/5
  invalid/1 -> can_valid_process/6
  setvalid/1 -> can_valid_process/7
  ovrdflg/1 -> can_valid_process/8
  ovrdval/1 -> can_valid_process/9
  srvcflg/1 -> can_valid_process/10
  srvcval/1 -> can_valid_process/11

== system inp/INP/CAN_Message_Valid_Proc/TCM2Valid ==
  signal_in : Inport
  Active_fault_flg : Inport  Port=2
  TRUE : Constant  Value=TRUE
  can_valid_process : inp/can_valid_process
  defval : Constant  Value=KINP_CANMsgValidTCM2_flg_defval
  invalid : Constant  Value=KINP_CANMsgValidTCM2_flg_invalid
  ovrdflg : Constant  Value=KINP_CANMsgValidTCM2_flg_ovrdflg
  ovrdval : Constant  Value=KINP_CANMsgValidTCM2_flg_ovrdval
  setvalid : Constant  Value=KINP_CANMsgValidTCM2_flg_setvalid
  srvcflg : Constant  Value=KINP_CANMsgValidTCM2_flg_srvcflg
  srvcval : Constant  Value=KINP_CANMsgValidTCM2_flg_srvcval
  tempval : Constant  Value=KINP_CANMsgValidTCM2_flg_tempval
  signal_out : Outport
  signal_valid_out : Outport  Port=2
  wires:
  srvcval/1 -> can_valid_process/11
  srvcflg/1 -> can_valid_process/10
  ovrdval/1 -> can_valid_process/9
  ovrdflg/1 -> can_valid_process/8
  setvalid/1 -> can_valid_process/7
  invalid/1 -> can_valid_process/6
  tempval/1 -> can_valid_process/5
  defval/1 -> can_valid_process/4
  TRUE/1 -> can_valid_process/3
  can_valid_process/1 -> signal_out/1
  can_valid_process/2 -> signal_valid_out/1
  signal_in/1 -> can_valid_process/1
  Active_fault_flg/1 -> can_valid_process/2

== system inp/INP/CAN_Message_Valid_Proc/VCU1Checked ==
  signal_in : Inport
  diagnosis_switch : Inport  Port=2
  Active_fault_flg : Inport  Port=3
  can_valid_process : inp/can_valid_process
  defval : Constant  Value=KINP_CANMsgCheckedVCU1_flg_defval
  invalid : Constant  Value=KINP_CANMsgCheckedVCU1_flg_invalid
  ovrdflg : Constant  Value=KINP_CANMsgCheckedVCU1_flg_ovrdflg
  ovrdval : Constant  Value=KINP_CANMsgCheckedVCU1_flg_ovrdval
  setvalid : Constant  Value=KINP_CANMsgCheckedVCU1_flg_setvalid
  srvcflg : Constant  Value=KINP_CANMsgCheckedVCU1_flg_srvcflg
  srvcval : Constant  Value=KINP_CANMsgCheckedVCU1_flg_srvcval
  tempval : Constant  Value=KINP_CANMsgCheckedVCU1_flg_tempval
  signal_out : Outport
  signal_valid_out : Outport  Port=2
  wires:
  can_valid_process/1 -> signal_out/1
  can_valid_process/2 -> signal_valid_out/1
  signal_in/1 -> can_valid_process/1
  Active_fault_flg/1 -> can_valid_process/2
  diagnosis_switch/1 -> can_valid_process/3
  defval/1 -> can_valid_process/4
  tempval/1 -> can_valid_process/5
  invalid/1 -> can_valid_process/6
  setvalid/1 -> can_valid_process/7
  ovrdflg/1 -> can_valid_process/8
  ovrdval/1 -> can_valid_process/9
  srvcflg/1 -> can_valid_process/10
  srvcval/1 -> can_valid_process/11

== system inp/INP/CAN_Message_Valid_Proc/VCU1Updated ==
  signal_in : Inport
  diagnosis_switch : Inport  Port=2
  Active_fault_flg : Inport  Port=3
  can_valid_process : inp/can_valid_process
  defval : Constant  Value=KINP_CANMsgUpdatedVCU1_flg_defval
  invalid : Constant  Value=KINP_CANMsgUpdatedVCU1_flg_invalid
  ovrdflg : Constant  Value=KINP_CANMsgUpdatedVCU1_flg_ovrdflg
  ovrdval : Constant  Value=KINP_CANMsgUpdatedVCU1_flg_ovrdval
  setvalid : Constant  Value=KINP_CANMsgUpdatedVCU1_flg_setvalid
  srvcflg : Constant  Value=KINP_CANMsgUpdatedVCU1_flg_srvcflg
  srvcval : Constant  Value=KINP_CANMsgUpdatedVCU1_flg_srvcval
  tempval : Constant  Value=KINP_CANMsgUpdatedVCU1_flg_tempval
  signal_out : Outport
  signal_valid_out : Outport  Port=2
  wires:
  srvcval/1 -> can_valid_process/11
  srvcflg/1 -> can_valid_process/10
  ovrdval/1 -> can_valid_process/9
  ovrdflg/1 -> can_valid_process/8
  setvalid/1 -> can_valid_process/7
  invalid/1 -> can_valid_process/6
  tempval/1 -> can_valid_process/5
  defval/1 -> can_valid_process/4
  diagnosis_switch/1 -> can_valid_process/3
  Active_fault_flg/1 -> can_valid_process/2
  signal_in/1 -> can_valid_process/1
  can_valid_process/2 -> signal_valid_out/1
  can_valid_process/1 -> signal_out/1

== system inp/INP/CAN_Message_Valid_Proc/VCU1Valid ==
  signal_in : Inport
  Active_fault_flg : Inport  Port=2
  TRUE : Constant  Value=TRUE
  can_valid_process : inp/can_valid_process
  defval : Constant  Value=KINP_CANMsgValidVCU1_flg_defval
  invalid : Constant  Value=KINP_CANMsgValidVCU1_flg_invalid
  ovrdflg : Constant  Value=KINP_CANMsgValidVCU1_flg_ovrdflg
  ovrdval : Constant  Value=KINP_CANMsgValidVCU1_flg_ovrdval
  setvalid : Constant  Value=KINP_CANMsgValidVCU1_flg_setvalid
  srvcflg : Constant  Value=KINP_CANMsgValidVCU1_flg_srvcflg
  srvcval : Constant  Value=KINP_CANMsgValidVCU1_flg_srvcval
  tempval : Constant  Value=KINP_CANMsgValidVCU1_flg_tempval
  signal_out : Outport
  signal_valid_out : Outport  Port=2
  wires:
  srvcval/1 -> can_valid_process/11
  srvcflg/1 -> can_valid_process/10
  ovrdval/1 -> can_valid_process/9
  ovrdflg/1 -> can_valid_process/8
  setvalid/1 -> can_valid_process/7
  invalid/1 -> can_valid_process/6
  tempval/1 -> can_valid_process/5
  defval/1 -> can_valid_process/4
  TRUE/1 -> can_valid_process/3
  Active_fault_flg/1 -> can_valid_process/2
  signal_in/1 -> can_valid_process/1
  can_valid_process/2 -> signal_valid_out/1
  can_valid_process/1 -> signal_out/1

== system inp/INP/CAN_Message_Valid_Proc/VCU2Checked ==
  signal_in : Inport
  diagnosis_switch : Inport  Port=2
  Active_fault_flg : Inport  Port=3
  can_valid_process : inp/can_valid_process
  defval : Constant  Value=KINP_CANMsgCheckedVCU2_flg_defval
  invalid : Constant  Value=KINP_CANMsgCheckedVCU2_flg_invalid
  ovrdflg : Constant  Value=KINP_CANMsgCheckedVCU2_flg_ovrdflg
  ovrdval : Constant  Value=KINP_CANMsgCheckedVCU2_flg_ovrdval
  setvalid : Constant  Value=KINP_CANMsgCheckedVCU2_flg_setvalid
  srvcflg : Constant  Value=KINP_CANMsgCheckedVCU2_flg_srvcflg
  srvcval : Constant  Value=KINP_CANMsgCheckedVCU2_flg_srvcval
  tempval : Constant  Value=KINP_CANMsgCheckedVCU2_flg_tempval
  signal_out : Outport
  signal_valid_out : Outport  Port=2
  wires:
  srvcval/1 -> can_valid_process/11
  srvcflg/1 -> can_valid_process/10
  ovrdval/1 -> can_valid_process/9
  ovrdflg/1 -> can_valid_process/8
  setvalid/1 -> can_valid_process/7
  invalid/1 -> can_valid_process/6
  tempval/1 -> can_valid_process/5
  defval/1 -> can_valid_process/4
  diagnosis_switch/1 -> can_valid_process/3
  Active_fault_flg/1 -> can_valid_process/2
  signal_in/1 -> can_valid_process/1
  can_valid_process/2 -> signal_valid_out/1
  can_valid_process/1 -> signal_out/1

== system inp/INP/CAN_Message_Valid_Proc/VCU2Updated ==
  signal_in : Inport
  diagnosis_switch : Inport  Port=2
  Active_fault_flg : Inport  Port=3
  can_valid_process : inp/can_valid_process
  defval : Constant  Value=KINP_CANMsgUpdatedVCU2_flg_defval
  invalid : Constant  Value=KINP_CANMsgUpdatedVCU2_flg_invalid
  ovrdflg : Constant  Value=KINP_CANMsgUpdatedVCU2_flg_ovrdflg
  ovrdval : Constant  Value=KINP_CANMsgUpdatedVCU2_flg_ovrdval
  setvalid : Constant  Value=KINP_CANMsgUpdatedVCU2_flg_setvalid
  srvcflg : Constant  Value=KINP_CANMsgUpdatedVCU2_flg_srvcflg
  srvcval : Constant  Value=KINP_CANMsgUpdatedVCU2_flg_srvcval
  tempval : Constant  Value=KINP_CANMsgUpdatedVCU2_flg_tempval
  signal_out : Outport
  signal_valid_out : Outport  Port=2
  wires:
  can_valid_process/1 -> signal_out/1
  can_valid_process/2 -> signal_valid_out/1
  signal_in/1 -> can_valid_process/1
  Active_fault_flg/1 -> can_valid_process/2
  diagnosis_switch/1 -> can_valid_process/3
  defval/1 -> can_valid_process/4
  tempval/1 -> can_valid_process/5
  invalid/1 -> can_valid_process/6
  setvalid/1 -> can_valid_process/7
  ovrdflg/1 -> can_valid_process/8
  ovrdval/1 -> can_valid_process/9
  srvcflg/1 -> can_valid_process/10
  srvcval/1 -> can_valid_process/11

== system inp/INP/CAN_Message_Valid_Proc/VCU2Valid ==
  signal_in : Inport
  Active_fault_flg : Inport  Port=2
  TRUE : Constant  Value=TRUE
  can_valid_process : inp/can_valid_process
  defval : Constant  Value=KINP_CANMsgValidVCU2_flg_defval
  invalid : Constant  Value=KINP_CANMsgValidVCU2_flg_invalid
  ovrdflg : Constant  Value=KINP_CANMsgValidVCU2_flg_ovrdflg
  ovrdval : Constant  Value=KINP_CANMsgValidVCU2_flg_ovrdval
  setvalid : Constant  Value=KINP_CANMsgValidVCU2_flg_setvalid
  srvcflg : Constant  Value=KINP_CANMsgValidVCU2_flg_srvcflg
  srvcval : Constant  Value=KINP_CANMsgValidVCU2_flg_srvcval
  tempval : Constant  Value=KINP_CANMsgValidVCU2_flg_tempval
  signal_out : Outport
  signal_valid_out : Outport  Port=2
  wires:
  can_valid_process/1 -> signal_out/1
  can_valid_process/2 -> signal_valid_out/1
  signal_in/1 -> can_valid_process/1
  Active_fault_flg/1 -> can_valid_process/2
  TRUE/1 -> can_valid_process/3
  defval/1 -> can_valid_process/4
  tempval/1 -> can_valid_process/5
  invalid/1 -> can_valid_process/6
  setvalid/1 -> can_valid_process/7
  ovrdflg/1 -> can_valid_process/8
  ovrdval/1 -> can_valid_process/9
  srvcflg/1 -> can_valid_process/10
  srvcval/1 -> can_valid_process/11

== system inp/INP/ECM_signals/AccelPedalPosn ==
  signal_in : Inport
  signal_valid_in : Inport  Port=2
  msg_valid : Inport  Port=3
  Active_fault_flg : Inport  Port=4
  can_signal_process_with_validity : inp/can_signal_process_with_validity
  conv_gain_offset : Subsystem
  defval : Constant  Value=KINP_AccelPedalPosn_pct_defval
  invalid : Constant  Value=KINP_AccelPedalPosn_pct_invalid
  min_max_limit : Subsystem
  ovrdflg : Constant  Value=KINP_AccelPedalPosn_pct_ovrdflg
  ovrdval : Constant  Value=KINP_AccelPedalPosn_pct_ovrdval
  setvalid : Constant  Value=KINP_AccelPedalPosn_pct_setvalid
  srvcflg : Constant  Value=KINP_AccelPedalPosn_pct_srvcflg
  srvcval : Constant  Value=KINP_AccelPedalPosn_pct_srvcval
  signal_out : Outport
  signal_valid_out : Outport  Port=2
  raw_signal_valid_out : Outport  Port=3
  wires:
  can_signal_process_with_validity/1 -> min_max_limit/1(in)
  can_signal_process_with_validity/2 -> signal_valid_out/1
  signal_in/1 -> conv_gain_offset/1(in)
  signal_valid_in/1 -> can_signal_process_with_validity/2
  msg_valid/1 -> can_signal_process_with_validity/3, can_signal_process_with_validity/5
  conv_gain_offset/1(out) -> can_signal_process_with_validity/1
  min_max_limit/1(out) -> signal_out/1
  Active_fault_flg/1 -> can_signal_process_with_validity/4
  can_signal_process_with_validity/3 -> raw_signal_valid_out/1
  defval/1 -> can_signal_process_with_validity/6
  invalid/1 -> can_signal_process_with_validity/7
  setvalid/1 -> can_signal_process_with_validity/8
  ovrdflg/1 -> can_signal_process_with_validity/9
  ovrdval/1 -> can_signal_process_with_validity/10
  srvcflg/1 -> can_signal_process_with_validity/11
  srvcval/1 -> can_signal_process_with_validity/12

== system inp/INP/ECM_signals/EMSHybridOpState ==
  signal_in : Inport
  msg_valid : Inport  Port=2
  Active_fault_flg : Inport  Port=3
  can_signal_process_wio_validity : inp/can_signal_process_wio_validity
  defval : Constant  Value=KINP_EMSHybridOpState_enum_defval
  invalid : Constant  Value=KINP_EMSHybridOpState_enum_invalid
  ovrdflg : Constant  Value=KINP_EMSHybridOpState_enum_ovrdflg
  ovrdval : Constant  Value=KINP_EMSHybridOpState_enum_ovrdval
  setvalid : Constant  Value=KINP_EMSHybridOpState_enum_setvalid
  srvcflg : Constant  Value=KINP_EMSHybridOpState_enum_srvcflg
  srvcval : Constant  Value=KINP_EMSHybridOpState_enum_srvcval
  signal_out : Outport
  signal_valid_out : Outport  Port=2
  wires:
  Active_fault_flg/1 -> can_signal_process_wio_validity/3
  srvcval/1 -> can_signal_process_wio_validity/10
  srvcflg/1 -> can_signal_process_wio_validity/9
  ovrdval/1 -> can_signal_process_wio_validity/8
  ovrdflg/1 -> can_signal_process_wio_validity/7
  setvalid/1 -> can_signal_process_wio_validity/6
  invalid/1 -> can_signal_process_wio_validity/5
  defval/1 -> can_signal_process_wio_validity/4
  msg_valid/1 -> can_signal_process_wio_validity/2
  signal_in/1 -> can_signal_process_wio_validity/1
  can_signal_process_wio_validity/2 -> signal_valid_out/1
  can_signal_process_wio_validity/1 -> signal_out/1

== system inp/INP/ECM_signals/EMSOpState ==
  signal_in : Inport
  msg_valid : Inport  Port=2
  Active_fault_flg : Inport  Port=3
  can_signal_process_wio_validity : inp/can_signal_process_wio_validity
  defval : Constant  Value=KINP_EMSOpState_enum_defval
  invalid : Constant  Value=KINP_EMSOpState_enum_invalid
  ovrdflg : Constant  Value=KINP_EMSOpState_enum_ovrdflg
  ovrdval : Constant  Value=KINP_EMSOpState_enum_ovrdval
  setvalid : Constant  Value=KINP_EMSOpState_enum_setvalid
  srvcflg : Constant  Value=KINP_EMSOpState_enum_srvcflg
  srvcval : Constant  Value=KINP_EMSOpState_enum_srvcval
  signal_out : Outport
  signal_valid_out : Outport  Port=2
  wires:
  can_signal_process_wio_validity/1 -> signal_out/1
  can_signal_process_wio_validity/2 -> signal_valid_out/1
  signal_in/1 -> can_signal_process_wio_validity/1
  msg_valid/1 -> can_signal_process_wio_validity/2
  defval/1 -> can_signal_process_wio_validity/4
  invalid/1 -> can_signal_process_wio_validity/5
  setvalid/1 -> can_signal_process_wio_validity/6
  ovrdflg/1 -> can_signal_process_wio_validity/7
  ovrdval/1 -> can_signal_process_wio_validity/8
  srvcflg/1 -> can_signal_process_wio_validity/9
  srvcval/1 -> can_signal_process_wio_validity/10
  Active_fault_flg/1 -> can_signal_process_wio_validity/3

== system inp/INP/ECM_signals/EffAccelPedalPosn ==
  signal_in : Inport
  signal_valid_in : Inport  Port=2
  msg_valid : Inport  Port=3
  Active_fault_flg : Inport  Port=4
  can_signal_process_with_validity : inp/can_signal_process_with_validity
  conv_gain_offset : Subsystem
  defval : Constant  Value=KINP_EffAccelPedalPosn_pct_defval
  invalid : Constant  Value=KINP_EffAccelPedalPosn_pct_invalid
  min_max_limit : Subsystem
  ovrdflg : Constant  Value=KINP_EffAccelPedalPosn_pct_ovrdflg
  ovrdval : Constant  Value=KINP_EffAccelPedalPosn_pct_ovrdval
  setvalid : Constant  Value=KINP_EffAccelPedalPosn_pct_setvalid
  srvcflg : Constant  Value=KINP_EffAccelPedalPosn_pct_srvcflg
  srvcval : Constant  Value=KINP_EffAccelPedalPosn_pct_srvcval
  signal_out : Outport
  signal_valid_out : Outport  Port=2
  raw_signal_valid_out : Outport  Port=3
  wires:
  srvcval/1 -> can_signal_process_with_validity/12
  srvcflg/1 -> can_signal_process_with_validity/11
  ovrdval/1 -> can_signal_process_with_validity/10
  ovrdflg/1 -> can_signal_process_with_validity/9
  setvalid/1 -> can_signal_process_with_validity/8
  invalid/1 -> can_signal_process_with_validity/7
  defval/1 -> can_signal_process_with_validity/6
  can_signal_process_with_validity/3 -> raw_signal_valid_out/1
  Active_fault_flg/1 -> can_signal_process_with_validity/4
  min_max_limit/1(out) -> signal_out/1
  conv_gain_offset/1(out) -> can_signal_process_with_validity/1
  msg_valid/1 -> can_signal_process_with_validity/5, can_signal_process_with_validity/3
  signal_valid_in/1 -> can_signal_process_with_validity/2
  signal_in/1 -> conv_gain_offset/1(in)
  can_signal_process_with_validity/2 -> signal_valid_out/1
  can_signal_process_with_validity/1 -> min_max_limit/1(in)

== system inp/INP/ECM_signals/EngHoodOpen ==
  signal_in : Inport
  msg_valid : Inport  Port=2
  Active_fault_flg : Inport  Port=3
  can_signal_process_wio_validity : inp/can_signal_process_wio_validity
  defval : Constant  Value=KINP_EngHoodOpen_flg_defval
  invalid : Constant  Value=KINP_EngHoodOpen_flg_invalid
  ovrdflg : Constant  Value=KINP_EngHoodOpen_flg_ovrdflg
  ovrdval : Constant  Value=KINP_EngHoodOpen_flg_ovrdval
  setvalid : Constant  Value=KINP_EngHoodOpen_flg_setvalid
  srvcflg : Constant  Value=KINP_EngHoodOpen_flg_srvcflg
  srvcval : Constant  Value=KINP_EngHoodOpen_flg_srvcval
  signal_out : Outport
  signal_valid_out : Outport  Port=2
  wires:
  Active_fault_flg/1 -> can_signal_process_wio_validity/3
  srvcval/1 -> can_signal_process_wio_validity/10
  srvcflg/1 -> can_signal_process_wio_validity/9
  ovrdval/1 -> can_signal_process_wio_validity/8
  ovrdflg/1 -> can_signal_process_wio_validity/7
  setvalid/1 -> can_signal_process_wio_validity/6
  invalid/1 -> can_signal_process_wio_validity/5
  defval/1 -> can_signal_process_wio_validity/4
  msg_valid/1 -> can_signal_process_wio_validity/2
  signal_in/1 -> can_signal_process_wio_validity/1
  can_signal_process_wio_validity/2 -> signal_valid_out/1
  can_signal_process_wio_validity/1 -> signal_out/1

== system inp/INP/ECM_signals/EngTqUnCORFault ==
  signal_in : Inport
  msg_valid : Inport  Port=2
  Active_fault_flg : Inport  Port=3
  can_signal_process_wio_validity : inp/can_signal_process_wio_validity
  defval : Constant  Value=KINP_EngTqUnCORFault_flg_defval
  invalid : Constant  Value=KINP_EngTqUnCORFault_flg_invalid
  ovrdflg : Constant  Value=KINP_EngTqUnCORFault_flg_ovrdflg
  ovrdval : Constant  Value=KINP_EngTqUnCORFault_flg_ovrdval
  setvalid : Constant  Value=KINP_EngTqUnCORFault_flg_setvalid
  srvcflg : Constant  Value=KINP_EngTqUnCORFault_flg_srvcflg
  srvcval : Constant  Value=KINP_EngTqUnCORFault_flg_srvcval
  signal_out : Outport
  signal_valid_out : Outport  Port=2
  wires:
  can_signal_process_wio_validity/1 -> signal_out/1
  can_signal_process_wio_validity/2 -> signal_valid_out/1
  signal_in/1 -> can_signal_process_wio_validity/1
  msg_valid/1 -> can_signal_process_wio_validity/2
  defval/1 -> can_signal_process_wio_validity/4
  invalid/1 -> can_signal_process_wio_validity/5
  setvalid/1 -> can_signal_process_wio_validity/6
  ovrdflg/1 -> can_signal_process_wio_validity/7
  ovrdval/1 -> can_signal_process_wio_validity/8
  srvcflg/1 -> can_signal_process_wio_validity/9
  srvcval/1 -> can_signal_process_wio_validity/10
  Active_fault_flg/1 -> can_signal_process_wio_validity/3

== system inp/INP/ECM_signals/EngineFrictionTorque ==
  signal_in : Inport
  signal_valid_in : Inport  Port=2
  msg_valid : Inport  Port=3
  Active_fault_flg : Inport  Port=4
  can_signal_process_with_validity : inp/can_signal_process_with_validity
  conv_gain_offset : Subsystem
  defval : Constant  Value=KINP_EngTqFriction_Nm_defval
  invalid : Constant  Value=KINP_EngTqFriction_Nm_invalid
  min_max_limit : Subsystem
  ovrdflg : Constant  Value=KINP_EngTqFriction_Nm_ovrdflg
  ovrdval : Constant  Value=KINP_EngTqFriction_Nm_ovrdval
  setvalid : Constant  Value=KINP_EngTqFriction_Nm_setvalid
  srvcflg : Constant  Value=KINP_EngTqFriction_Nm_srvcflg
  srvcval : Constant  Value=KINP_EngTqFriction_Nm_srvcval
  signal_out : Outport
  signal_valid_out : Outport  Port=2
  raw_signal_valid_out : Outport  Port=3
  wires:
  srvcval/1 -> can_signal_process_with_validity/12
  srvcflg/1 -> can_signal_process_with_validity/11
  ovrdval/1 -> can_signal_process_with_validity/10
  ovrdflg/1 -> can_signal_process_with_validity/9
  setvalid/1 -> can_signal_process_with_validity/8
  invalid/1 -> can_signal_process_with_validity/7
  defval/1 -> can_signal_process_with_validity/6
  can_signal_process_with_validity/3 -> raw_signal_valid_out/1
  Active_fault_flg/1 -> can_signal_process_with_validity/4
  min_max_limit/1(out) -> signal_out/1
  conv_gain_offset/1(out) -> can_signal_process_with_validity/1
  msg_valid/1 -> can_signal_process_with_validity/5, can_signal_process_with_validity/3
  signal_valid_in/1 -> can_signal_process_with_validity/2
  signal_in/1 -> conv_gain_offset/1(in)
  can_signal_process_with_validity/2 -> signal_valid_out/1
  can_signal_process_with_validity/1 -> min_max_limit/1(in)
